simulink model "BaseEngineController_A02_bak"  (Simulink 7.7)
settings: Solver=FixedStepDiscrete  StartTime=0.0  StopTime=10.0  RelTol=1e-3
PreLoadFcn:
  | try, eval([bdroot '_setup']); catch, eval('model_setup', ''); end
InitFcn:
  | try, eval([bdroot '_setup']); catch, eval('model_setup', ''); end
undefined symbols (not defined in the file or in its folder): ECU555, High, motohawk_build_version, motohawk_get_faults, motohawk_trunc_name, motohawk_vardec_config_group, motohawk_vardec_path, True
libraries: MotoHawk_lib x1145, AutoDoc_lib x786, motohawk_control_primitives_lib x114, motohawk_engine_lib x19, motohawk_annotations_lib x12, simulink x11, simulink_extras x1
(part 7 of 23)

== system BaseEngineController_A02_bak/Foreground/Control/VirtualSensors/RawProcessed ==
  Raw : Inport
  Indexes : Inport  Port=2
  APP Sensor Management : Subsystem
      mask Display:
      | patch([0 0 1 1], [0 0.075 0.075 0], [230/255 0/255 0/255])
      | patch([0 0 1 1], [0.025 0.05 0.05 0.025], [150/255 0/255 0/255])
      | patch([0 0 1 1], [0 0.025 0.025 0], [0/255 0/255 0/255])
      | 
      | disp('APP Sensor\nArbitration');
  Base TPS Request : Subsystem  TreatAsAtomicUnit=on
  Bus Creator : BusCreator  Inputs=2
  Bus Creator2 : BusCreator  Inputs=15
  Bus Selector1 : BusSelector  OutputSignals=PotSensors.TPS2
  Bus Selector10 : BusSelector  OutputSignals=PotSensors.TPS1
  Bus Selector11 : BusSelector  OutputSignals=NonLinearSensors.O2_PostCat
  Bus Selector12 : BusSelector  OutputSignals=RPM
  Bus Selector2 : BusSelector  OutputSignals=RPMInst
  Bus Selector3 : BusSelector  OutputSignals=LinearSensors.FuelPres,LinearSensors.Map_Time
  Bus Selector4 : BusSelector  OutputSignals=PotSensors.APP1,PotSensors.APP2
  Bus Selector5 : BusSelector  OutputSignals=NonLinearSensors.ECT_Raw
  Bus Selector6 : BusSelector  OutputSignals=TPS09Idx
  Bus Selector7 : BusSelector  OutputSignals=NonLinearSensors.IAT_Raw
  Capture ECT At Startup : Subsystem  TreatAsAtomicUnit=on  mask(Capture Signal At Startup){nam='ECT_KeyUp', count=5, units='C', grp='Sensors | ECT'}
      mask prompts: nam "Signal Name"; count "Number Of Samples To Average"; grp "MotoTune GroupString Name"
      mask init:
      | lbl = sprintf('CAPTURE IN STALL\nAverage of\nfirst %d samples', count);
      mask Display:
      | patch([0 0 1 1], [0 0.075 0.075 0], [230/255 0/255 0/255])
      | patch([0 0 1 1], [0.025 0.05 0.05 0.025], [150/255 0/255 0/255])
      | patch([0 0 1 1], [0 0.025 0.025 0], [0/255 0/255 0/255])
      | 
      | disp(lbl);
  Capture IAT At Startup : Subsystem  TreatAsAtomicUnit=on  mask(Capture Signal At Startup){nam='IAT_KeyUp', count=5, units='C', grp='Sensors | IAT'}
      mask prompts: nam "Signal Name"; count "Number Of Samples To Average"; grp "MotoTune GroupString Name"
      mask init:
      | lbl = sprintf('CAPTURE IN STALL\nAverage of\nfirst %d samples', count);
      mask Display:
      | patch([0 0 1 1], [0 0.075 0.075 0], [230/255 0/255 0/255])
      | patch([0 0 1 1], [0.025 0.05 0.05 0.025], [150/255 0/255 0/255])
      | patch([0 0 1 1], [0 0.025 0.025 0], [0/255 0/255 0/255])
      | 
      | disp(lbl);
  CutThrottle APP Limiting : Subsystem
      mask Display:
      | patch([0 0 1 1], [0 0.075 0.075 0], [230/255 0/255 0/255])
      | patch([0 0 1 1], [0.025 0.05 0.05 0.025], [150/255 0/255 0/255])
      | patch([0 0 1 1], [0 0.025 0.025 0], [0/255 0/255 0/255])
      | 
      | disp('');
  Documentation_Calibrations_Probes_Block : AutoDoc_lib/Documentation_Calibrations_Probes_Block
  Documentation_Left_Border : AutoDoc_lib/Documentation_Left_Border  layout=Portrait
  Documentation_Right_Border : AutoDoc_lib/Documentation_Right_Border  layout=Portrait
  Documentation_Title_Block : AutoDoc_lib/Documentation_Title_Block
  Engine Accel : Subsystem
  EngineState : Subsystem
      mask Display: disp('ENGINE STATE\nDETERMINATION\n\n1 - Stall\n2 - Crank\n3 - Run');
  Fuel Delta Pressure Calculation : Subsystem
      mask Display:
      | patch([0 0 1 1], [0 0.075 0.075 0], [230/255 0/255 0/255])
      | patch([0 0 1 1], [0.025 0.05 0.05 0.025], [150/255 0/255 0/255])
      | patch([0 0 1 1], [0 0.025 0.025 0], [0/255 0/255 0/255])
      | 
      | disp('Fuel Pressure \n Across Injectors');
  O2 De-lag : Subsystem
  Stall : motohawk_control_primitives_lib/CompareTo  SystemSampleTime=-1  op===  val=1
  TPS  Sensor Arbitration : Subsystem
      mask Display:
      | patch([0 0 1 1], [0 0.075 0.075 0], [230/255 0/255 0/255])
      | patch([0 0 1 1], [0.025 0.05 0.05 0.025], [150/255 0/255 0/255])
      | patch([0 0 1 1], [0 0.025 0.025 0], [0/255 0/255 0/255])
      | 
      | disp('TPS Sensor\nArbitration');
  Terminator : Terminator
  Time Since Key On : Subsystem
  Time Since Run : Subsystem
  Time Since Stall : Subsystem
  motohawk_data_read : MotoHawk_lib/Data Storage Blocks/motohawk_data_read  source=Lookup By Name  structure=Scalar  operation=Read entire data structure at once  sz_vec=4  sz_row=2  sz_col=3  typ=uint8  nam='EngineState'  checkNull=on  struct_inst_name='Struct1'  struct_nam='MyStruct'
  motohawk_probe : MotoHawk_lib/Calibration & Probing Blocks/motohawk_probe  nam='APPPcnt'  show_more=on  name_source=Use Parameter  read_level=1  view_as=Number  enum_struct={'Disabled', 'Enabled'}  vector_style=Wide Row  use_help_units=on  help='APP used in controller (after power curve and CutThrottle limiting'  units='%'  use_header_enum=off  row_header_enum={}  col_header_enum={}  use_precision=on  precision=''  gain=1  offset=0  exp=1  use_mototune_group=on  mototune_group=motohawk_vardec_path(gcb)
  RawProcessed : Outport
  wires:
  Base TPS Request/3(APP Idx) -> Bus Creator2/6  "APPIdx"
  Bus Selector7/1 -> Capture IAT At Startup/1(Signal_In)  "<IAT_Raw>"
  Capture IAT At Startup/1(Signal_KeyUp) -> Bus Creator2/15  "IAT_KeyUp"
  Bus Selector2/1 -> EngineState/1(RPM)  "<RPMInst>"
  Bus Selector4/1 -> APP Sensor Management/1(APP1)  "<APP1>"
  Bus Selector12/1 -> Engine Accel/1(RPM)  "<RPM>"
  EngineState/1(State) -> Bus Creator2/1  "EngineState"
  Base TPS Request/1(ETCReqFilt) -> Bus Creator2/4  "ETCRequest"
  Engine Accel/1(RPM Accel) -> Bus Creator2/9  "RPM_Accel"
  Bus Creator2/1 -> RawProcessed/1  "RawProcessed"
  Raw/1 -> Bus Selector11/1, Bus Selector12/1, Bus Selector10/1, Bus Selector7/1, Bus Selector5/1, Bus Selector1/1, Bus Selector3/1, Bus Selector4/1, Bus Selector2/1
  Bus Selector10/1 -> TPS  Sensor Arbitration/1(TPS1)  "<TPS1>"
  Bus Selector1/1 -> TPS  Sensor Arbitration/2(TPS2)  "<TPS2>"
  TPS  Sensor Arbitration/1(TPS) -> Bus Creator2/10  "TPS"
  Bus Selector5/1 -> Capture ECT At Startup/1(Signal_In)  "<ECT_Raw>"
  motohawk_data_read/1 -> Stall/1
  Stall/1 -> Capture IAT At Startup/Enable, Capture ECT At Startup/Enable
  Capture ECT At Startup/1(Signal_KeyUp) -> Bus Creator2/11  "ECT_KeyUp"
  Base TPS Request/2(ETCReqRaw) -> Bus Creator2/5  "ETCReqRaw"
  Time Since Stall/1(Time) -> Bus Creator2/12  "TimeSinceStall"
  Time Since Run/1(Time) -> Bus Creator2/13  "TimeSinceRun"
  APP Sensor Management/2(APP_Raw) -> Bus Creator2/3, CutThrottle APP Limiting/1(APP_in [%])  "APP_Raw"
  Bus Selector6/1 -> Base TPS Request/2(TPS_Idx)  "<TPS09Idx>"
  Indexes/1 -> Bus Selector6/1
  Time Since Key On/1(Time) -> Bus Creator2/14  "TimeSinceKeyOn"
  Bus Selector11/1 -> O2 De-lag/2(PostCat), O2 De-lag/1(PreCat)  "<O2_PostCat>"
  Bus Creator/1 -> Bus Creator2/8  "O2Delagged"
  O2 De-lag/1(PreCatDelagged) -> Bus Creator/1  "PreO2Delag"
  O2 De-lag/2(PostCatDelagged) -> Bus Creator/2  "PostO2Delag"
  Bus Selector3/1 -> Fuel Delta Pressure Calculation/1(FuelPressure)  "<FuelPres>"
  Bus Selector3/2 -> Fuel Delta Pressure Calculation/2("MAP ")  "<Map_Time>"
  Fuel Delta Pressure Calculation/1("FuelDeltaPressure ") -> Bus Creator2/7  "FuelDeltaPressure"
  Bus Selector4/2 -> ?  "<APP2>"
  CutThrottle APP Limiting/1(APP_out [%]) -> Bus Creator2/2, Base TPS Request/1(APP Pcnt), motohawk_probe/1  "APPPcnt"
  APP Sensor Management/1(APP_out) -> Terminator/1

== system BaseEngineController_A02_bak/Foreground/Control/VirtualSensors/Virtual Indexes ==
  TorqueCalcs : Inport
  AirCalcs : Inport  Port=2
  RawProcessed : Inport  Port=3
  Bus Creator2 : BusCreator  Inputs=9
  Bus Selector : BusSelector  OutputSignals=Torque
  Bus Selector1 : BusSelector  OutputSignals=Power
  Bus Selector2 : BusSelector  OutputSignals=NECCT
  Bus Selector3 : BusSelector  OutputSignals=MAFPort
  Bus Selector4 : BusSelector  OutputSignals=ECT_KeyUp
  Bus Selector5 : BusSelector  OutputSignals=RPM_Accel
  Bus Selector6 : BusSelector  OutputSignals=APPPcnt
  Documentation_Calibrations_Probes_Block : AutoDoc_lib/Documentation_Calibrations_Probes_Block
  Documentation_Left_Border : AutoDoc_lib/Documentation_Left_Border  layout=Portrait
  Documentation_Right_Border : AutoDoc_lib/Documentation_Right_Border  layout=Portrait
  Documentation_Title_Block : AutoDoc_lib/Documentation_Title_Block
  ECT_KeyUp : Subsystem
  MAFPortIndex : Subsystem
  NECCT Index : Subsystem
  Power Index : Subsystem
  Torque Indexes : Subsystem
  motohawk_prelookup : MotoHawk_lib/Lookup Tables/motohawk_prelookup  nam='RPMAccel17'  breakpoint_data=ones(1,17)  show_more=on  data_type=Inherit from 'Table Data'  storage=Constant  read_level=1  write_level=1  ovr_cals=on  use_help=on  help=''  units=''  use_min_max=off  min_val=-inf  max_val=inf  use_precision=on  precision=''  gain=1  offset=0  exp=1  use_links=off  input_name=''  use_mototune_group=on  mototune_group=motohawk_vardec_path(gcb)
  motohawk_prelookup1 : MotoHawk_lib/Lookup Tables/motohawk_prelookup  nam='App05'  breakpoint_data=[0 50 70 90 100]  show_more=on  data_type=Inherit from 'Table Data'  storage=Constant  read_level=1  write_level=1  ovr_cals=on  use_help=on  help=''  units=''  use_min_max=off  min_val=-inf  max_val=inf  use_precision=on  precision=''  gain=1  offset=0  exp=1  use_links=off  input_name=''  use_mototune_group=on  mototune_group=motohawk_vardec_path(gcb)
  VirtualIndexes : Outport
  wires:
  Torque Indexes/3(IndTorqueNom17Idx) -> Bus Creator2/3  "IndTorqueNom17Idx"
  MAFPortIndex/1(MAFPort05Idx) -> Bus Creator2/6  "MAFPort05Idx"
  NECCT Index/1(NECCT09Idx) -> Bus Creator2/5  "NECCT09Idx"
  Power Index/1(IndPower05Idx) -> Bus Creator2/4  "IndPower05Idx"
  Torque Indexes/2(IndTorqueNom09Idx) -> Bus Creator2/2  "IndTorqueNom09Idx"
  Torque Indexes/1(IndTorqueNom05Idx) -> Bus Creator2/1  "IndTorqueNom05Idx"
  Bus Creator2/1 -> VirtualIndexes/1  "Virtual Indexes"
  TorqueCalcs/1 -> Bus Selector1/1, Bus Selector2/1, Bus Selector/1
  Bus Selector/1 -> Torque Indexes/1(EngineTorque)  "<Torque>"
  Bus Selector1/1 -> Power Index/1(EnginePower)  "<Power>"
  Bus Selector2/1 -> NECCT Index/1(NECCT)  "<NECCT>"
  AirCalcs/1 -> Bus Selector3/1
  Bus Selector3/1 -> MAFPortIndex/1(MAFPort)  "<MAFPort>"
  ECT_KeyUp/1(ECT_KeyUp09Idx) -> Bus Creator2/7  "ECTKeyUp09Idx"
  Bus Selector4/1 -> ECT_KeyUp/1(ECT_KeyUp)  "<ECT_KeyUp>"
  RawProcessed/1 -> Bus Selector4/1, Bus Selector5/1, Bus Selector6/1
  motohawk_prelookup/1 -> Bus Creator2/8  "RPMAccel17Idx"
  Bus Selector5/1 -> motohawk_prelookup/1  "<RPM_Accel>"
  Bus Selector6/1 -> motohawk_prelookup1/1  "<APPPcnt>"
  motohawk_prelookup1/1 -> Bus Creator2/9  "App05Idx"

== system BaseEngineController_A02_bak/Foreground/Inputs/CAN Inputs/112 ==
  Bus Creator : BusCreator  Inputs=3
  ESTOP at Startup : Subsystem
  Logical Operator : Logic  Operator=NOT  AllPortsSameDT=off  OutDataTypeStr=boolean
  Logical Operator1 : Logic  Operator=XOR  AllPortsSameDT=off  OutDataTypeStr=boolean
  Read CAN Message : MotoHawk_lib/CAN Blocks/Read CAN Message  nam='CAN_1'  bus=-2  msg=CANRx_112_210  queue=1  slot=''  valid=off  stale=off  labelwires=off
  motohawk_data_def : MotoHawk_lib/Data Storage Blocks/motohawk_data_def  nam='ESTOP'  container_nam=''  data=0  typ=boolean  struct_nam='MyStruct'  storage=Volatile  outputRef=off  use_vardec=on  window=Display  read_level=1  write_level=1  ovr_cals=on  view_as=Number  enum_struct={'Disabled', 'Enabled'}  vector_style=Wide Row  use_help_units=off  help=''  units=''  use_header_enum=off  row_header_enum=''  col_header_enum=''  use_min_max=off  min_val=-inf  max_val=inf  use_precision=off  precision=''  gain=1  offset=0  exp=1  use_mototune_group=off  mototune_group=motohawk_vardec_path(gcb)  eval_name=MyData  data_stores=motohawk_sfun_data_def_data_stores  struct_instances=motohawk_sfun_data_def_struct_instances  vardecs=motohawk_sfun_data_def_vardecs
  motohawk_data_write : MotoHawk_lib/Data Storage Blocks/motohawk_data_write  source=Lookup By Name  structure=Scalar  operation=Write entire data structure at once  sz_vec=9  sz_row=2  sz_col=9  typ=boolean  nam='ESTOP'  checkNull=on  write_read_only=off  struct_inst_name=''  struct_name='MyStruct'
  motohawk_override_abs : MotoHawk_lib/Calibration & Probing Blocks/motohawk_override_abs  SystemSampleTime=-1  parent_name='Genset_Enable_Ovr'  parent_val=0  show_more=off  name_source=Use Parameter  parent_read_level=1  parent_override_level=1  view_as=Number  enum_struct={'Disabled', 'Enabled'}  use_help_units=off  parent_help=''  parent_units=''  use_min_max=off  parent_min=-inf  parent_max=inf  use_precision=off  parent_precision=''  parent_gain=1  parent_offset=0  parent_exp=1  use_mototune_group=off  parent_group=motohawk_vardec_path(gcb)
  motohawk_override_abs1 : MotoHawk_lib/Calibration & Probing Blocks/motohawk_override_abs  SystemSampleTime=-1  parent_name='Genset_Load_Ovr'  parent_val=0  show_more=off  name_source=Use Parameter  parent_read_level=1  parent_override_level=1  view_as=Number  enum_struct={'Disabled', 'Enabled'}  use_help_units=off  parent_help=''  parent_units=''  use_min_max=off  parent_min=-inf  parent_max=inf  use_precision=off  parent_precision=''  parent_gain=1  parent_offset=0  parent_exp=1  use_mototune_group=off  parent_group=motohawk_vardec_path(gcb)
  motohawk_override_abs2 : MotoHawk_lib/Calibration & Probing Blocks/motohawk_override_abs  SystemSampleTime=-1  parent_name='Vehicle_Speed_Ovr'  parent_val=0  show_more=off  name_source=Use Parameter  parent_read_level=1  parent_override_level=1  view_as=Number  enum_struct={'Disabled', 'Enabled'}  use_help_units=off  parent_help=''  parent_units=''  use_min_max=off  parent_min=-inf  parent_max=inf  use_precision=off  parent_precision=''  parent_gain=1  parent_offset=0  parent_exp=1  use_mototune_group=off  parent_group=motohawk_vardec_path(gcb)
  motohawk_probe : MotoHawk_lib/Calibration & Probing Blocks/motohawk_probe  nam='Genset_Enable_Prb'  show_more=off  name_source=Use Parameter  read_level=1  view_as=Number  enum_struct={'Disabled', 'Enabled'}  vector_style=Wide Row  use_help_units=off  help=''  units=''  use_header_enum=off  row_header_enum={}  col_header_enum={}  use_precision=off  precision=''  gain=1  offset=0  exp=1  use_mototune_group=off  mototune_group=motohawk_vardec_path(gcb)
  motohawk_probe1 : MotoHawk_lib/Calibration & Probing Blocks/motohawk_probe  nam='Genset_Load_Prb'  show_more=off  name_source=Use Parameter  read_level=1  view_as=Number  enum_struct={'Disabled', 'Enabled'}  vector_style=Wide Row  use_help_units=off  help=''  units=''  use_header_enum=off  row_header_enum={}  col_header_enum={}  use_precision=off  precision=''  gain=1  offset=0  exp=1  use_mototune_group=off  mototune_group=motohawk_vardec_path(gcb)
  motohawk_probe2 : MotoHawk_lib/Calibration & Probing Blocks/motohawk_probe  nam='Vehicle_Speed_Prb'  show_more=off  name_source=Use Parameter  read_level=1  view_as=Number  enum_struct={'Disabled', 'Enabled'}  vector_style=Wide Row  use_help_units=off  help=''  units=''  use_header_enum=off  row_header_enum={}  col_header_enum={}  use_precision=off  precision=''  gain=1  offset=0  exp=1  use_mototune_group=off  mototune_group=motohawk_vardec_path(gcb)
  Out1 : Outport
  wires:
  Read CAN Message/1 -> motohawk_override_abs/1, motohawk_probe/1  "Genset Enable"
  Bus Creator/1 -> Out1/1
  motohawk_override_abs/1 -> Bus Creator/1, Logical Operator/1  "Genset Enable"
  Read CAN Message/2 -> motohawk_override_abs1/1, motohawk_probe1/1  "Genset Enable"
  motohawk_override_abs1/1 -> Bus Creator/2  "Load Enable"
  Read CAN Message/3 -> motohawk_override_abs2/1, motohawk_probe2/1  "Genset Enable"
  motohawk_override_abs2/1 -> Bus Creator/3  "Vehicle Speed"
  ESTOP at Startup/1(ON [bool]) -> Logical Operator1/1
  Logical Operator1/1 -> motohawk_data_write/1
  Logical Operator/1 -> Logical Operator1/2
